simulink model "simulation_environment_thesis"  (R2024b)
settings: Solver=VariableStepAuto  StartTime=0.0  StopTime=3200  RelTol=1e-3
values from neighbouring files (symbols this model uses):
  A = [1, dt; 0, 1]    [estimate_and_control_fully_actuated_with_current.m]
  A1 = [1, 0, 0, 0, dt, 0 ... (first row only)    [estimate_and_control_fully_actuated_with_current.m]
  alpha = 10    [estimate_and_control_fully_actuated_with_current.m]
  alpha_xy_1 = 1    [estimate_and_control_fully_actuated_with_current.m]
  alpha_z_1 = 5    [estimate_and_control_fully_actuated_with_current.m]
  Az1 = [1, dt ... (first row only)    [estimate_and_control_fully_actuated_with_current.m]
  B = [dt; 0]    [estimate_and_control_fully_actuated_with_current.m]
  B1 = [dt, 0 ... (first row only)    [estimate_and_control_fully_actuated_with_current.m]
  Bz1 = [dt ... (first row only)    [estimate_and_control_fully_actuated_with_current.m]
  C1 = [1, 0, 0, 0, 0, 0 ... (first row only)    [estimate_and_control_fully_actuated_with_current.m]
  circumference_x = min_dist_from_ds*cos(theta)    [estimate_and_control_fully_actuated_with_current.m]
  circumference_y = min_dist_from_ds*sin(theta)    [estimate_and_control_fully_actuated_with_current.m]
  const_current = [-r; -s; 0; 0]    [estimate_and_control_fully_actuated_with_current.m]
  csi_psi_1 = 0.0003    [estimate_and_control_fully_actuated_with_current.m]
  csi_r_1 = 0.0003    [estimate_and_control_fully_actuated_with_current.m]
  csi_vcx_1 = 0.0003    [estimate_and_control_fully_actuated_with_current.m]
  csi_vcy_1 = 0.0003    [estimate_and_control_fully_actuated_with_current.m]
  csi_vcz_1 = 0.005    [estimate_and_control_fully_actuated_with_current.m]
  csi_x_1 = 0.003    [estimate_and_control_fully_actuated_with_current.m]
  csi_y_1 = 0.003    [estimate_and_control_fully_actuated_with_current.m]
  csi_z_1 = 0.005    [estimate_and_control_fully_actuated_with_current.m]
  Cz1 = [1, 0]    [estimate_and_control_fully_actuated_with_current.m]
  d = 1    [estimate_and_control_fully_actuated_with_current.m]
  delta_FOV = pi/7    (= 0.448798950513)    [estimate_and_control_fully_actuated_with_current.m]
  desired_speed = speed_0    [estimate_and_control_fully_actuated_with_current.m]
  dt = 1/Tsim    (= 0.005)    [estimate_and_control_fully_actuated_with_current.m]
  eta1 = deg2rad(0.5)    [estimate_and_control_fully_actuated_with_current.m]
  eta2 = deg2rad(0.5)    [estimate_and_control_fully_actuated_with_current.m]
  eta_psi_1 = 0.003    [estimate_and_control_fully_actuated_with_current.m]
  eta_x_1 = 0.03    [estimate_and_control_fully_actuated_with_current.m]
  eta_y_1 = 0.03    [estimate_and_control_fully_actuated_with_current.m]
  eta_z_1 = 0.005    [estimate_and_control_fully_actuated_with_current.m]
  G = dt*eye(2)    [estimate_and_control_fully_actuated_with_current.m]
  G1 = dt*eye(6)    [estimate_and_control_fully_actuated_with_current.m]
  Gz1 = dt*eye(2)    [estimate_and_control_fully_actuated_with_current.m]
  left_reference = L_x*[cos(NED_psi_ds); sin(NED_psi_ds); 0] + L_y_l*[-sin(NED_psi_ds); cos(NED_psi_ds); 0]    [estimate_and_control_fully_actuated_with_current.m]
  max_dist_from_ship = 60    [estimate_and_control_fully_actuated_with_current.m]
  min_dist_from_ds = 45    [estimate_and_control_fully_actuated_with_current.m]
  mu = -5    [estimate_and_control_fully_actuated_with_current.m]
  NED_psi_ds = -pi/2    (= -1.57079632679)    [estimate_and_control_fully_actuated_with_current.m]
  p_b1 = [-90; 90; 5]    [estimate_and_control_fully_actuated_with_current.m]
  position_t_0 = [0; 0; 0; 0]    [estimate_and_control_fully_actuated_with_current.m]
  psi_P = linspace(0,2*pi,360)'    [estimate_and_control_fully_actuated_with_current.m]
  Q = [csi1_kf^2, 0 ... (first row only)    [estimate_and_control_fully_actuated_with_current.m]
  Q1 = [csi_x_1_kf^2, 0, 0, 0, 0, 0 ... (first row only)    [estimate_and_control_fully_actuated_with_current.m]
  Qz1 = [csi_z_1_kf^2, 0 ... (first row only)    [estimate_and_control_fully_actuated_with_current.m]
  R = [eta1_kf^2, 0 ... (first row only)    [estimate_and_control_fully_actuated_with_current.m]
  R1 = [eta_x_1_kf^2, 0, 0 ... (first row only)    [estimate_and_control_fully_actuated_with_current.m]
  right_reference = L_x*[cos(NED_psi_ds); sin(NED_psi_ds); 0] + L_y_r*[sin(NED_psi_ds); -cos(NED_psi_ds); 0]    [estimate_and_control_fully_actuated_with_current.m]
  Rz1 = eta_z_1_kf^2    (= 1e-06)    [estimate_and_control_fully_actuated_with_current.m]
  speed_0 = [0.1, 0.1, 0, 0]'    [estimate_and_control_fully_actuated_with_current.m]
  tau_heave_sat = 30    [estimate_and_control_fully_actuated_with_current.m]
  tau_surge_sat = 10    [estimate_and_control_fully_actuated_with_current.m]
  tau_sway_sat = 30    [estimate_and_control_fully_actuated_with_current.m]
  tau_yaw_sat = 0.1    [estimate_and_control_fully_actuated_with_current.m]
  var_vm_x = 0.001    [estimate_and_control_fully_actuated_with_current.m]
  var_vm_y = 0.001    [estimate_and_control_fully_actuated_with_current.m]
  var_vm_z = 0.001    [estimate_and_control_fully_actuated_with_current.m]
undefined symbols (not defined in the file or in its folder): A2, alpha2, B2, csi1_2, csi2_2, csi3_2, csi4_2, csi5_2, csi6_2, csi7_2, csi8_2, eta1_2, eta2_2, eta3_2, eta4_2, eta5_2, eta6_2, G2, Q2, R2
libraries: simulink x60, simulink_extras x5
(part 5 of 16)

== system simulation_environment_thesis/PHASE 2.21/dynamic + kinematic models4 ==
  note: body frame
  note: ds frame
  note: body frame
  tau : Inport
  ref speed : Inport  Port=2
  last_yaw__from_ship : Inport  Port=3
  yaw_est : Inport  Port=4
  ds_p_auv : Inport  Port=5
  Constant : Constant  Value=0
  Demux : Demux
  Integrator1 : Integrator  InitialConditionSource=external
  Integrator2 : Integrator  InitialConditionSource=external
  Integrator4 : Integrator  InitialCondition=speed_0  UpperSaturationLimit=10^3  LowerSaturationLimit=-10^3
  Integrator5 : Integrator  InitialCondition=const_current
  MATLAB Function : MATLAB Function  TreatAsAtomicUnit=on
  MATLAB Function7 : MATLAB Function  TreatAsAtomicUnit=on
  MATLAB Function9 : MATLAB Function  TreatAsAtomicUnit=on
  Mux : Mux  Inputs=2
  Mux1 : Mux  Inputs=2
  Mux3 : Mux  Inputs=3
  Mux4 : Mux
  Random Number : RandomNumber  Variance=csi1_2^2  Seed=290635734  SampleTime=dt
  Random Number1 : RandomNumber  Variance=csi3_2^2  Seed=1610892866  SampleTime=dt
  Random Number10 : RandomNumber  Variance=csi8_2^2  Seed=308002504  SampleTime=dt
  Random Number3 : RandomNumber  Variance=csi6_2^2  Seed=137035395  SampleTime=dt
  Random Number4 : RandomNumber  Variance=csi4_2^2  Seed=690147537  SampleTime=dt
  Random Number5 : RandomNumber  Variance=csi2_2^2  Seed=1826908498  SampleTime=dt
  Random Number8 : RandomNumber  Variance=csi5_2^2  Seed=1139063427  SampleTime=dt
  Random Number9 : RandomNumber  Variance=csi7_2^2  Seed=54081086  SampleTime=dt
  Selector1 : Selector  InputPortWidth=4  IndexOptions=Index vector (dialog)  Indices=[4]  OutputSizes=1
  Selector2 : Selector  InputPortWidth=4  IndexOptions=Index vector (dialog)  Indices=[1 2 3]  OutputSizes=1
  Selector3 : Selector  InputPortWidth=4  IndexOptions=Index vector (dialog)  Indices=[1 2 3]  OutputSizes=1
  Sum{,1..2,5} (4 alike) : Sum  Inputs=|++
  Sum4 : Sum  Inputs=|+-
  error speed : Outport
  v_tot ds : Outport  Port=2
  p : Outport  Port=3
  yaw : Outport  Port=4
  body speed : Outport  Port=5
  wires:
  ref speed/1 -> Sum4/1
  MATLAB Function9/1(eta_dot) -> Sum5/1, Demux/1
  tau/1 -> MATLAB Function7/2(tau)
  Sum4/1 -> error speed/1
  Sum5/1 -> Selector3/1, v_tot ds/1
  Integrator4/1 -> MATLAB Function7/1(speed), Sum4/2, body speed/1, MATLAB Function9/2(speed)
  Integrator5/1 -> Sum5/2
  last_yaw__from_ship/1 -> Integrator1/2
  Integrator1/1 -> MATLAB Function/1("angle ")
  yaw_est/1 -> MATLAB Function9/1(yaw)
  MATLAB Function/1(angle) -> Mux/2, yaw/1
  ds_p_auv/1 -> Integrator2/2
  Random Number1/1 -> Mux3/3
  Mux3/1 -> Sum/2
  Sum/1 -> Integrator2/1
  Random Number5/1 -> Mux3/2
  Random Number/1 -> Mux3/1
  Integrator2/1 -> Mux/1
  Random Number10/1 -> Sum2/2
  Selector1/1 -> Sum2/1
  Selector2/1 -> Mux1/1
  Sum2/1 -> Mux1/2
  Mux1/1 -> Integrator4/1
  MATLAB Function7/1(speed_dot) -> Selector2/1, Selector1/1
  Random Number9/1 -> Sum1/2
  Sum1/1 -> Integrator1/1
  Demux/4 -> Sum1/1
  Random Number8/1 -> Mux4/2
  Random Number4/1 -> Mux4/1
  Mux4/1 -> Integrator5/1
  Random Number3/1 -> Mux4/3
  Selector3/1 -> Sum/1
  Mux/1 -> p/1
  Constant/1 -> Mux4/4

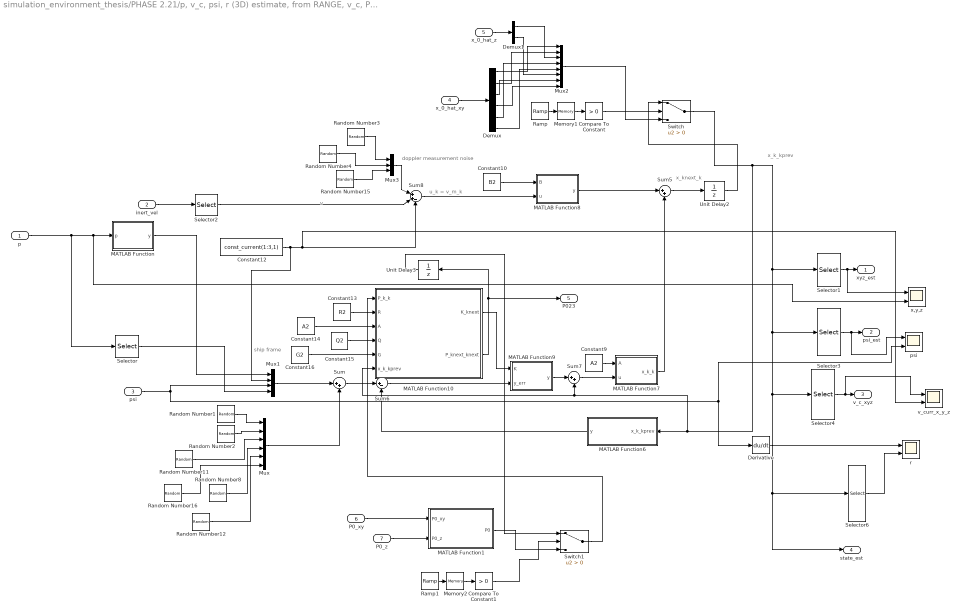
== system simulation_environment_thesis/PHASE 2.21/p, v_c, psi, r (3D) estimate, from RANGE, v_c, PSI measurement ==
  note: x_k_kprev
  note: x_knext_k
  note: ship frame
  note: v
  note: doppler measurement noise
  note: u_k = v_m_k
  p : Inport
  inert_vel : Inport  Port=2
  psi : Inport  Port=3
  x_0_hat_xy : Inport  Port=4
  x_0_hat_z : Inport  Port=5
  P0_xy : Inport  Port=6
  P0_z : Inport  Port=7
  Compare To Constant : simulink/Logic and Bit Operations/Compare To Constant  relop=>  const=0  OutDataTypeStr=boolean
  Compare To Constant1 : simulink/Logic and Bit Operations/Compare To Constant  relop=>  const=0  OutDataTypeStr=boolean
  Constant10 : Constant  Value=B2
  Constant12 : Constant  Value=const_current(1:3,1)  VectorParams1D=off
  Constant13 : Constant  Value=R2
  Constant14 : Constant  Value=A2
  Constant15 : Constant  Value=Q2
  Constant16 : Constant  Value=G2
  Constant9 : Constant  Value=A2  VectorParams1D=off
  Demux : Demux  Outputs=6
  Demux1 : Demux  Outputs=2
  Derivative : Derivative
  MATLAB Function : MATLAB Function  TreatAsAtomicUnit=on
  MATLAB Function1 : MATLAB Function  TreatAsAtomicUnit=on
  MATLAB Function10 : MATLAB Function  TreatAsAtomicUnit=on
  MATLAB Function6 : MATLAB Function  TreatAsAtomicUnit=on
  MATLAB Function7 : MATLAB Function  TreatAsAtomicUnit=on
  MATLAB Function8 : MATLAB Function  TreatAsAtomicUnit=on
  MATLAB Function9 : MATLAB Function  TreatAsAtomicUnit=on
  Memory1 : Memory
  Memory2 : Memory
  Mux : Mux  Inputs=6
  Mux1 : Mux
  Mux2 : Mux  Inputs=8
  Mux3 : Mux  Inputs=3
  Ramp : simulink/Sources/Ramp  slope=1  start=0  InitialOutput=0
  Ramp1 : simulink/Sources/Ramp  slope=1  start=0  InitialOutput=0
  Random Number1 : RandomNumber  Variance=eta1_2^2  Seed=1592011725  SampleTime=dt
  Random Number11 : RandomNumber  Variance=eta3_2^2  Seed=403145296  SampleTime=dt
  Random Number12 : RandomNumber  Variance=eta5_2^2  Seed=421479706  SampleTime=dt
  Random Number15 : RandomNumber  Variance=var_vm_z^2  Seed=536323856  SampleTime=dt
  Random Number16 : RandomNumber  Variance=eta6_2^2  Seed=285935395  SampleTime=dt
  Random Number2 : RandomNumber  Variance=eta2_2^2  Seed=1745211012  SampleTime=dt
  Random Number3 : RandomNumber  Variance=var_vm_x^2  Seed=980211847  SampleTime=dt
  Random Number4 : RandomNumber  Variance=var_vm_y^2  Seed=1399358789  SampleTime=dt
  Random Number8 : RandomNumber  Variance=eta4_2^2  Seed=2011049955  SampleTime=dt
  Selector : Selector  InputPortWidth=3  IndexOptions=Index vector (dialog)  Indices=[3]  OutputSizes=1
  Selector1 : Selector  InputPortWidth=8  IndexOptions=Index vector (dialog)  Indices=[1 2 3]  OutputSizes=1
  Selector2 : Selector  InputPortWidth=4  IndexOptions=Index vector (dialog)  Indices=[1 2 3]  OutputSizes=1
  Selector3 : Selector  InputPortWidth=8  IndexOptions=Index vector (dialog)  Indices=[7]  OutputSizes=1
  Selector4 : Selector  InputPortWidth=8  IndexOptions=Index vector (dialog)  Indices=[4 5 6]  OutputSizes=1
  Selector6 : Selector  InputPortWidth=8  IndexOptions=Index vector (dialog)  Indices=[8]  OutputSizes=1
  Sum : Sum  Inputs=|++
  Sum5 : Sum  Inputs=|++
  Sum6 : Sum  Inputs=|+-
  Sum7 : Sum  Inputs=|++
  Sum8 : Sum  Inputs=|++-
  Switch : Switch  Criteria=u2 > Threshold  SaturateOnIntegerOverflow=off  InputSameDT=off
  Switch1 : Switch  Criteria=u2 > Threshold  SaturateOnIntegerOverflow=off  InputSameDT=off
  Unit Delay2 : UnitDelay  SampleTime=-1  HasFrameUpgradeWarning=on
  Unit Delay3 : UnitDelay  InitialCondition=alpha2*eye(8)  SampleTime=-1  HasFrameUpgradeWarning=on
  "psi " : Scope  NumInputPorts=2
  r : Scope  NumInputPorts=2
  v_curr_x_y_z : Scope  NumInputPorts=2
  x,y,z : Scope  NumInputPorts=2
  xyz_est : Outport
  psi_est : Outport  Port=2
  v_c_xyz : Outport  Port=3
  state_est : Outport  Port=4
  P023 : Outport  Port=5
  wires:
  MATLAB Function7/1(x_k_k) -> Sum5/2
  Constant14/1 -> MATLAB Function10/3(A)
  Sum7/1 -> MATLAB Function7/2(u)
  Random Number4/1 -> Mux3/2
  MATLAB Function8/1(y) -> Sum5/1
  Random Number3/1 -> Mux3/1
  Constant15/1 -> MATLAB Function10/4(Q)
  MATLAB Function9/1(y) -> Sum7/1
  Sum5/1 -> Unit Delay2/1
  MATLAB Function10/2(P_knext_knext) -> P023/1, Unit Delay3/1
  Sum8/1 -> MATLAB Function8/2(u)
  MATLAB Function10/1(K_knext) -> MATLAB Function9/1(K)
  Constant9/1 -> MATLAB Function7/1(A)
  Constant13/1 -> MATLAB Function10/2(R)
  Sum6/1 -> MATLAB Function9/2(y_err)
  Constant10/1 -> MATLAB Function8/1(B)
  Mux3/1 -> Sum8/1
  Constant16/1 -> MATLAB Function10/5(G)
  inert_vel/1 -> Selector2/1
  Sum/1 -> Sum6/1
  MATLAB Function6/1(y) -> Sum6/2
  Selector1/1 -> x,y,z/1, xyz_est/1
  psi/1 -> "psi "/2, Derivative/1, Mux1/3
  Mux1/1 -> Sum/1
  Selector3/1 -> "psi "/1, psi_est/1
  Selector4/1 -> v_curr_x_y_z/1, v_c_xyz/1
  Selector2/1 -> Sum8/2
  Mux/1 -> Sum/2
  Random Number2/1 -> Mux/2
  Random Number11/1 -> Mux/3
  Random Number1/1 -> Mux/1
  MATLAB Function/1(y) -> Mux1/1
  Constant12/1 -> Mux1/2, Sum8/3, v_curr_x_y_z/2
  Random Number8/1 -> Mux/4
  Random Number12/1 -> Mux/5
  Derivative/1 -> r/1
  Selector6/1 -> r/2
  Random Number15/1 -> Mux3/3
  Random Number16/1 -> Mux/6
  Selector/1 -> Mux1/4
  p/1 -> Selector/1, x,y,z/2, MATLAB Function/1(p)
  Switch/1 -> Selector3/1, Selector4/1, state_est/1, Selector6/1, Selector1/1, MATLAB Function10/6(x_k_kprev), Sum7/2, MATLAB Function6/1(x_k_kprev)
  Memory1/1 -> Compare To Constant/1
  Ramp/1 -> Memory1/1
  Compare To Constant/1 -> Switch/2
  Unit Delay2/1 -> Switch/1
  x_0_hat_z/1 -> Demux1/1
  x_0_hat_xy/1 -> Demux/1
  Demux/1 -> Mux2/1
  Demux/2 -> Mux2/2
  Demux1/1 -> Mux2/3
  Demux/5 -> Mux2/4
  Demux/6 -> Mux2/5
  Demux1/2 -> Mux2/6
  Demux/3 -> Mux2/7
  Demux/4 -> Mux2/8
  Mux2/1 -> Switch/3
  P0_xy/1 -> MATLAB Function1/1(P0_xy)
  P0_z/1 -> MATLAB Function1/2(P0_z)
  Ramp1/1 -> Memory2/1
  Memory2/1 -> Compare To Constant1/1
  Compare To Constant1/1 -> Switch1/2
  MATLAB Function1/1(P0) -> Switch1/3
  Unit Delay3/1 -> Switch1/1
  Switch1/1 -> MATLAB Function10/1(P_k_k)

== system simulation_environment_thesis/PHASE 2.22/CONTROLLER4 ==
  v_err : Inport
  psi_err : Inport  Port=2
  speed_0 : Inport  Port=3
  z_err : Inport  Port=4
  ANTIWINDUP SURGE : Subsystem
  ANTIWINDUP SWAY : Subsystem
  ANTIWINDUP_HEAVE : Subsystem
  ANTIWINDUP_YAW : Subsystem
  Demux : Demux
  MATLAB Function3 : MATLAB Function  TreatAsAtomicUnit=on
  Mux : Mux
  tau : Outport
  wires:
  MATLAB Function3/12(K_i_psi) -> ANTIWINDUP_YAW/1(K1)
  ANTIWINDUP SURGE/1(tau_u) -> Mux/1
  ANTIWINDUP_HEAVE/1(tau_w) -> Mux/3
  ANTIWINDUP_YAW/1(tau_psi) -> Mux/4
  MATLAB Function3/1(K_i_u) -> ANTIWINDUP SURGE/2(K)
  MATLAB Function3/3(K_t_u) -> ANTIWINDUP SURGE/4(K_t_u)
  MATLAB Function3/2(K_p_u) -> ANTIWINDUP SURGE/3(K1)
  speed_0/1 -> MATLAB Function3/1(speed_0)
  Demux/1 -> ANTIWINDUP SURGE/1(in)
  MATLAB Function3/14(K_t_psi) -> ANTIWINDUP_YAW/3(K_t_psi)
  MATLAB Function3/11(K_p_psi) -> ANTIWINDUP_YAW/2(K2)
  ANTIWINDUP SWAY/1(tau_v) -> Mux/2
  v_err/1 -> Demux/1
  Mux/1 -> tau/1
  psi_err/1 -> ANTIWINDUP_YAW/4(in1)
  Demux/2 -> ANTIWINDUP SWAY/1(in)
  MATLAB Function3/4(K_i_v) -> ANTIWINDUP SWAY/2(K)
  MATLAB Function3/5(K_p_v) -> ANTIWINDUP SWAY/3(K1)
  MATLAB Function3/6(K_t_v) -> ANTIWINDUP SWAY/4(K_t_v)
  MATLAB Function3/13(K_d_psi) -> ANTIWINDUP_YAW/6(K)
  Demux/4 -> ANTIWINDUP_YAW/5(in)
  MATLAB Function3/7(K_i_w) -> ANTIWINDUP_HEAVE/1(K1)
  MATLAB Function3/8(K_p_w) -> ANTIWINDUP_HEAVE/2(K2)
  MATLAB Function3/9(K_d_w) -> ANTIWINDUP_HEAVE/6(K)
  MATLAB Function3/10(K_t_w) -> ANTIWINDUP_HEAVE/3(K_t_w)
  Demux/3 -> ANTIWINDUP_HEAVE/5(in)
  z_err/1 -> ANTIWINDUP_HEAVE/4(in1)

== system simulation_environment_thesis/PHASE 2.22/dynamic + kinematic models1 ==
  note: body frame
  note: ds frame
  note: body frame
  tau : Inport
  ref speed : Inport  Port=2
  last_yaw__from_ship : Inport  Port=3
  yaw_est : Inport  Port=4
  ds_p_auv : Inport  Port=5
  Constant : Constant  Value=0
  Demux : Demux
  Integrator1 : Integrator  InitialConditionSource=external
  Integrator2 : Integrator  InitialConditionSource=external
  Integrator4 : Integrator  InitialCondition=speed_0  UpperSaturationLimit=10^3  LowerSaturationLimit=-10^3
  Integrator5 : Integrator  InitialCondition=const_current
  MATLAB Function : MATLAB Function  TreatAsAtomicUnit=on
  MATLAB Function7 : MATLAB Function  TreatAsAtomicUnit=on
  MATLAB Function9 : MATLAB Function  TreatAsAtomicUnit=on
  Mux : Mux  Inputs=2
  Mux1 : Mux  Inputs=2
  Mux3 : Mux  Inputs=3
  Mux4 : Mux
  Random Number : RandomNumber  Variance=csi1_2^2  Seed=1280987953  SampleTime=dt
  Random Number1 : RandomNumber  Variance=csi3_2^2  Seed=123915637  SampleTime=dt
  Random Number10 : RandomNumber  Variance=csi8_2^2  Seed=337781319  SampleTime=dt
  Random Number3 : RandomNumber  Variance=csi6_2^2  Seed=1365143274  SampleTime=dt
  Random Number4 : RandomNumber  Variance=csi4_2^2  Seed=894166265  SampleTime=dt
  Random Number5 : RandomNumber  Variance=csi2_2^2  Seed=538465485  SampleTime=dt
  Random Number8 : RandomNumber  Variance=csi5_2^2  Seed=774913930  SampleTime=dt
  Random Number9 : RandomNumber  Variance=csi7_2^2  Seed=364931984  SampleTime=dt
  Selector1 : Selector  InputPortWidth=4  IndexOptions=Index vector (dialog)  Indices=[4]  OutputSizes=1
  Selector2 : Selector  InputPortWidth=4  IndexOptions=Index vector (dialog)  Indices=[1 2 3]  OutputSizes=1
  Selector3 : Selector  InputPortWidth=4  IndexOptions=Index vector (dialog)  Indices=[1 2 3]  OutputSizes=1
  Sum{,1..2,5} (4 alike) : Sum  Inputs=|++
  Sum4 : Sum  Inputs=|+-
  error speed : Outport
  v_tot ds : Outport  Port=2
  p : Outport  Port=3
  yaw : Outport  Port=4
  body speed : Outport  Port=5
  wires:
  ref speed/1 -> Sum4/1
  MATLAB Function9/1(eta_dot) -> Sum5/1, Demux/1
  tau/1 -> MATLAB Function7/2(tau)
  Sum4/1 -> error speed/1
  Sum5/1 -> Selector3/1, v_tot ds/1
  Integrator4/1 -> MATLAB Function7/1(speed), Sum4/2, body speed/1, MATLAB Function9/2(speed)
  Integrator5/1 -> Sum5/2
  last_yaw__from_ship/1 -> Integrator1/2
  Integrator1/1 -> MATLAB Function/1("angle ")
  yaw_est/1 -> MATLAB Function9/1(yaw)
  MATLAB Function/1(angle) -> Mux/2, yaw/1
  ds_p_auv/1 -> Integrator2/2
  Random Number1/1 -> Mux3/3
  Mux3/1 -> Sum/2
  Sum/1 -> Integrator2/1
  Random Number5/1 -> Mux3/2
  Random Number/1 -> Mux3/1
  Integrator2/1 -> Mux/1
  Random Number10/1 -> Sum2/2
  Selector1/1 -> Sum2/1
  Selector2/1 -> Mux1/1
  Sum2/1 -> Mux1/2
  Mux1/1 -> Integrator4/1
  MATLAB Function7/1(speed_dot) -> Selector2/1, Selector1/1
  Random Number9/1 -> Sum1/2
  Sum1/1 -> Integrator1/1
  Demux/4 -> Sum1/1
  Random Number8/1 -> Mux4/2
  Random Number4/1 -> Mux4/1
  Mux4/1 -> Integrator5/1
  Random Number3/1 -> Mux4/3
  Selector3/1 -> Sum/1
  Mux/1 -> p/1
  Constant/1 -> Mux4/4

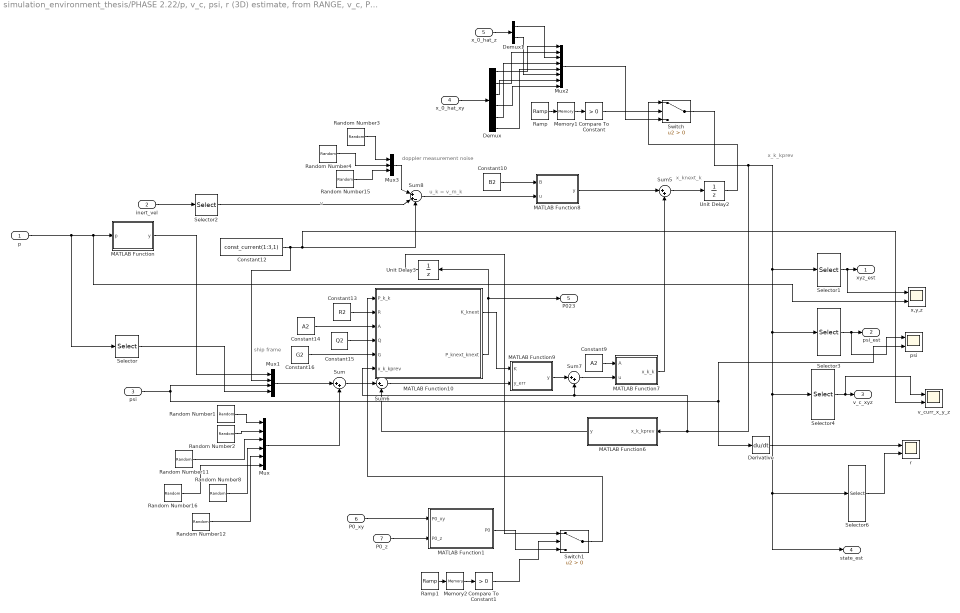
== system simulation_environment_thesis/PHASE 2.22/p, v_c, psi, r (3D) estimate, from RANGE, v_c, PSI measurement ==
  note: x_k_kprev
  note: x_knext_k
  note: ship frame
  note: v
  note: doppler measurement noise
  note: u_k = v_m_k
  p : Inport
  inert_vel : Inport  Port=2
  psi : Inport  Port=3
  x_0_hat_xy : Inport  Port=4
  x_0_hat_z : Inport  Port=5
  P0_xy : Inport  Port=6
  P0_z : Inport  Port=7
  Compare To Constant : simulink/Logic and Bit Operations/Compare To Constant  relop=>  const=0  OutDataTypeStr=boolean
  Compare To Constant1 : simulink/Logic and Bit Operations/Compare To Constant  relop=>  const=0  OutDataTypeStr=boolean
  Constant10 : Constant  Value=B2
  Constant12 : Constant  Value=const_current(1:3,1)  VectorParams1D=off
  Constant13 : Constant  Value=R2
  Constant14 : Constant  Value=A2
  Constant15 : Constant  Value=Q2
  Constant16 : Constant  Value=G2
  Constant9 : Constant  Value=A2  VectorParams1D=off
  Demux : Demux  Outputs=6
  Demux1 : Demux  Outputs=2
  Derivative : Derivative
  MATLAB Function : MATLAB Function  TreatAsAtomicUnit=on
  MATLAB Function1 : MATLAB Function  TreatAsAtomicUnit=on
  MATLAB Function10 : MATLAB Function  TreatAsAtomicUnit=on
  MATLAB Function6 : MATLAB Function  TreatAsAtomicUnit=on
  MATLAB Function7 : MATLAB Function  TreatAsAtomicUnit=on
  MATLAB Function8 : MATLAB Function  TreatAsAtomicUnit=on
  MATLAB Function9 : MATLAB Function  TreatAsAtomicUnit=on
  Memory1 : Memory
  Memory2 : Memory
  Mux : Mux  Inputs=6
  Mux1 : Mux
  Mux2 : Mux  Inputs=8
  Mux3 : Mux  Inputs=3
  Ramp : simulink/Sources/Ramp  slope=1  start=0  InitialOutput=0
  Ramp1 : simulink/Sources/Ramp  slope=1  start=0  InitialOutput=0
  Random Number1 : RandomNumber  Variance=eta1_2^2  Seed=1089217264  SampleTime=dt
  Random Number11 : RandomNumber  Variance=eta3_2^2  Seed=72035191  SampleTime=dt
  Random Number12 : RandomNumber  Variance=eta5_2^2  Seed=2079342519  SampleTime=dt
  Random Number15 : RandomNumber  Variance=var_vm_z^2  Seed=110850037  SampleTime=dt
  Random Number16 : RandomNumber  Variance=eta6_2^2  Seed=1575761613  SampleTime=dt
  Random Number2 : RandomNumber  Variance=eta2_2^2  Seed=1411597288  SampleTime=dt
  Random Number3 : RandomNumber  Variance=var_vm_x^2  Seed=1818164200  SampleTime=dt
  Random Number4 : RandomNumber  Variance=var_vm_y^2  Seed=1838379340  SampleTime=dt
  Random Number8 : RandomNumber  Variance=eta4_2^2  Seed=1668214198  SampleTime=dt
  Selector : Selector  InputPortWidth=3  IndexOptions=Index vector (dialog)  Indices=[3]  OutputSizes=1
  Selector1 : Selector  InputPortWidth=8  IndexOptions=Index vector (dialog)  Indices=[1 2 3]  OutputSizes=1
  Selector2 : Selector  InputPortWidth=4  IndexOptions=Index vector (dialog)  Indices=[1 2 3]  OutputSizes=1
  Selector3 : Selector  InputPortWidth=8  IndexOptions=Index vector (dialog)  Indices=[7]  OutputSizes=1
  Selector4 : Selector  InputPortWidth=8  IndexOptions=Index vector (dialog)  Indices=[4 5 6]  OutputSizes=1
  Selector6 : Selector  InputPortWidth=8  IndexOptions=Index vector (dialog)  Indices=[8]  OutputSizes=1
  Sum : Sum  Inputs=|++
  Sum5 : Sum  Inputs=|++
  Sum6 : Sum  Inputs=|+-
  Sum7 : Sum  Inputs=|++
  Sum8 : Sum  Inputs=|++-
  Switch : Switch  Criteria=u2 > Threshold  SaturateOnIntegerOverflow=off  InputSameDT=off
  Switch1 : Switch  Criteria=u2 > Threshold  SaturateOnIntegerOverflow=off  InputSameDT=off
  Unit Delay2 : UnitDelay  SampleTime=-1  HasFrameUpgradeWarning=on
  Unit Delay3 : UnitDelay  InitialCondition=alpha2*eye(8)  SampleTime=-1  HasFrameUpgradeWarning=on
  "psi " : Scope  NumInputPorts=2
  r : Scope  NumInputPorts=2
  v_curr_x_y_z : Scope  NumInputPorts=2
  x,y,z : Scope  NumInputPorts=2
  xyz_est : Outport
  psi_est : Outport  Port=2
  v_c_xyz : Outport  Port=3
  state_est : Outport  Port=4
  P023 : Outport  Port=5
  wires:
  MATLAB Function7/1(x_k_k) -> Sum5/2
  Constant14/1 -> MATLAB Function10/3(A)
  Sum7/1 -> MATLAB Function7/2(u)
  Random Number4/1 -> Mux3/2
  MATLAB Function8/1(y) -> Sum5/1
  Random Number3/1 -> Mux3/1
  Constant15/1 -> MATLAB Function10/4(Q)
  MATLAB Function9/1(y) -> Sum7/1
  Sum5/1 -> Unit Delay2/1
  MATLAB Function10/2(P_knext_knext) -> P023/1, Unit Delay3/1
  Sum8/1 -> MATLAB Function8/2(u)
  MATLAB Function10/1(K_knext) -> MATLAB Function9/1(K)
  Constant9/1 -> MATLAB Function7/1(A)
  Constant13/1 -> MATLAB Function10/2(R)
  Sum6/1 -> MATLAB Function9/2(y_err)
  Constant10/1 -> MATLAB Function8/1(B)
  Mux3/1 -> Sum8/1
  Constant16/1 -> MATLAB Function10/5(G)
  inert_vel/1 -> Selector2/1
  Sum/1 -> Sum6/1
  MATLAB Function6/1(y) -> Sum6/2
  Selector1/1 -> x,y,z/1, xyz_est/1
  psi/1 -> "psi "/2, Derivative/1, Mux1/3
  Mux1/1 -> Sum/1
  Selector3/1 -> "psi "/1, psi_est/1
  Selector4/1 -> v_curr_x_y_z/1, v_c_xyz/1
  Selector2/1 -> Sum8/2
  Mux/1 -> Sum/2
  Random Number2/1 -> Mux/2
  Random Number11/1 -> Mux/3
  Random Number1/1 -> Mux/1
  MATLAB Function/1(y) -> Mux1/1
  Constant12/1 -> Mux1/2, Sum8/3, v_curr_x_y_z/2
  Random Number8/1 -> Mux/4
  Random Number12/1 -> Mux/5
  Derivative/1 -> r/1
  Selector6/1 -> r/2
  Random Number15/1 -> Mux3/3
  Random Number16/1 -> Mux/6
  Selector/1 -> Mux1/4
  p/1 -> Selector/1, x,y,z/2, MATLAB Function/1(p)
  Switch/1 -> Selector3/1, Selector4/1, state_est/1, Selector6/1, Selector1/1, Sum7/2, MATLAB Function10/6(x_k_kprev), MATLAB Function6/1(x_k_kprev)
  Memory1/1 -> Compare To Constant/1
  Ramp/1 -> Memory1/1
  Compare To Constant/1 -> Switch/2
  Unit Delay2/1 -> Switch/1
  x_0_hat_z/1 -> Demux1/1
  x_0_hat_xy/1 -> Demux/1
  Demux/1 -> Mux2/1
  Demux/2 -> Mux2/2
  Demux1/1 -> Mux2/3
  Demux/5 -> Mux2/4
  Demux/6 -> Mux2/5
  Demux1/2 -> Mux2/6
  Demux/3 -> Mux2/7
  Demux/4 -> Mux2/8
  Mux2/1 -> Switch/3
  P0_xy/1 -> MATLAB Function1/1(P0_xy)
  P0_z/1 -> MATLAB Function1/2(P0_z)
  Ramp1/1 -> Memory2/1
  Memory2/1 -> Compare To Constant1/1
  Compare To Constant1/1 -> Switch1/2
  MATLAB Function1/1(P0) -> Switch1/3
  Unit Delay3/1 -> Switch1/1
  Switch1/1 -> MATLAB Function10/1(P_k_k)

== system simulation_environment_thesis/PHASE 2.24/CONTROLLER4 ==
  v_err : Inport
  psi_err : Inport  Port=2
  speed_0 : Inport  Port=3
  z_err : Inport  Port=4
  ANTIWINDUP SURGE : Subsystem
  ANTIWINDUP SWAY : Subsystem
  ANTIWINDUP_HEAVE : Subsystem
  ANTIWINDUP_YAW : Subsystem
  Demux : Demux
  MATLAB Function3 : MATLAB Function  TreatAsAtomicUnit=on
  Mux : Mux
  tau : Outport
  wires:
  MATLAB Function3/12(K_i_psi) -> ANTIWINDUP_YAW/1(K1)
  ANTIWINDUP SURGE/1(tau_u) -> Mux/1
  ANTIWINDUP_HEAVE/1(tau_w) -> Mux/3
  ANTIWINDUP_YAW/1(tau_psi) -> Mux/4
  MATLAB Function3/1(K_i_u) -> ANTIWINDUP SURGE/2(K)
  MATLAB Function3/3(K_t_u) -> ANTIWINDUP SURGE/4(K_t_u)
  MATLAB Function3/2(K_p_u) -> ANTIWINDUP SURGE/3(K1)
  speed_0/1 -> MATLAB Function3/1(speed_0)
  Demux/1 -> ANTIWINDUP SURGE/1(in)
  MATLAB Function3/14(K_t_psi) -> ANTIWINDUP_YAW/3(K_t_psi)
  MATLAB Function3/11(K_p_psi) -> ANTIWINDUP_YAW/2(K2)
  ANTIWINDUP SWAY/1(tau_v) -> Mux/2
  v_err/1 -> Demux/1
  Mux/1 -> tau/1
  psi_err/1 -> ANTIWINDUP_YAW/4(in1)
  Demux/2 -> ANTIWINDUP SWAY/1(in)
  MATLAB Function3/4(K_i_v) -> ANTIWINDUP SWAY/2(K)
  MATLAB Function3/5(K_p_v) -> ANTIWINDUP SWAY/3(K1)
  MATLAB Function3/6(K_t_v) -> ANTIWINDUP SWAY/4(K_t_v)
  MATLAB Function3/13(K_d_psi) -> ANTIWINDUP_YAW/6(K)
  Demux/4 -> ANTIWINDUP_YAW/5(in)
  MATLAB Function3/7(K_i_w) -> ANTIWINDUP_HEAVE/1(K1)
  MATLAB Function3/8(K_p_w) -> ANTIWINDUP_HEAVE/2(K2)
  MATLAB Function3/9(K_d_w) -> ANTIWINDUP_HEAVE/6(K)
  MATLAB Function3/10(K_t_w) -> ANTIWINDUP_HEAVE/3(K_t_w)
  Demux/3 -> ANTIWINDUP_HEAVE/5(in)
  z_err/1 -> ANTIWINDUP_HEAVE/4(in1)

== system simulation_environment_thesis/PHASE 2.24/dynamic + kinematic models1 ==
  note: body frame
  note: ds frame
  note: body frame
  tau : Inport
  ref speed : Inport  Port=2
  last_yaw__from_ship : Inport  Port=3
  yaw_est : Inport  Port=4
  ds_p_auv : Inport  Port=5
  Constant : Constant  Value=0
  Demux : Demux
  Integrator1 : Integrator  InitialConditionSource=external
  Integrator2 : Integrator  InitialConditionSource=external
  Integrator4 : Integrator  InitialCondition=speed_0  UpperSaturationLimit=10^3  LowerSaturationLimit=-10^3
  Integrator5 : Integrator  InitialCondition=const_current
  MATLAB Function : MATLAB Function  TreatAsAtomicUnit=on
  MATLAB Function7 : MATLAB Function  TreatAsAtomicUnit=on
  MATLAB Function9 : MATLAB Function  TreatAsAtomicUnit=on
  Mux : Mux  Inputs=2
  Mux1 : Mux  Inputs=2
  Mux3 : Mux  Inputs=3
  Mux4 : Mux
  Random Number : RandomNumber  Variance=csi_x_1^2  Seed=1833923873  SampleTime=dt
  Random Number1 : RandomNumber  Variance=csi_z_1^2  Seed=744874454  SampleTime=dt
  Random Number10 : RandomNumber  Variance=csi_r_1^2  Seed=863987044  SampleTime=dt
  Random Number3 : RandomNumber  Variance=csi_vcz_1^2  Seed=327348082  SampleTime=dt
  Random Number4 : RandomNumber  Variance=csi_vcx_1^2  Seed=1447632064  SampleTime=dt
  Random Number5 : RandomNumber  Variance=csi_y_1^2  Seed=1607275602  SampleTime=dt
  Random Number8 : RandomNumber  Variance=csi_vcy_1^2  Seed=1677010297  SampleTime=dt
  Random Number9 : RandomNumber  Variance=csi_psi_1^2  Seed=1596755842  SampleTime=dt
  Selector1 : Selector  InputPortWidth=4  IndexOptions=Index vector (dialog)  Indices=[4]  OutputSizes=1
  Selector2 : Selector  InputPortWidth=4  IndexOptions=Index vector (dialog)  Indices=[1 2 3]  OutputSizes=1
  Selector3 : Selector  InputPortWidth=4  IndexOptions=Index vector (dialog)  Indices=[1 2 3]  OutputSizes=1
  Sum{,1..2,5} (4 alike) : Sum  Inputs=|++
  Sum4 : Sum  Inputs=|+-
  error speed : Outport
  v_tot ds : Outport  Port=2
  body speed : Outport  Port=3
  p : Outport  Port=4
  yaw : Outport  Port=5
  wires:
  ref speed/1 -> Sum4/1
  MATLAB Function9/1(eta_dot) -> Sum5/1, Demux/1
  tau/1 -> MATLAB Function7/2(tau)
  Sum4/1 -> error speed/1
  Sum5/1 -> Selector3/1, v_tot ds/1
  Integrator4/1 -> MATLAB Function7/1(speed), Sum4/2, body speed/1, MATLAB Function9/2(speed)
  Integrator5/1 -> Sum5/2
  last_yaw__from_ship/1 -> Integrator1/2
  Integrator1/1 -> MATLAB Function/1("angle ")
  yaw_est/1 -> MATLAB Function9/1(yaw)
  MATLAB Function/1(angle) -> Mux/2, yaw/1
  ds_p_auv/1 -> Integrator2/2
  Random Number1/1 -> Mux3/3
  Mux3/1 -> Sum/2
  Sum/1 -> Integrator2/1
  Random Number5/1 -> Mux3/2
  Random Number/1 -> Mux3/1
  Integrator2/1 -> Mux/1
  Random Number10/1 -> Sum2/2
  Selector1/1 -> Sum2/1
  Selector2/1 -> Mux1/1
  Sum2/1 -> Mux1/2
  Mux1/1 -> Integrator4/1
  MATLAB Function7/1(speed_dot) -> Selector2/1, Selector1/1
  Random Number9/1 -> Sum1/2
  Sum1/1 -> Integrator1/1
  Demux/4 -> Sum1/1
  Random Number8/1 -> Mux4/2
  Random Number4/1 -> Mux4/1
  Mux4/1 -> Integrator5/1
  Random Number3/1 -> Mux4/3
  Selector3/1 -> Sum/1
  Mux/1 -> p/1
  Constant/1 -> Mux4/4

== system simulation_environment_thesis/PHASE 2.24/positon and current estimate, XY PLANE from X, Y, psi measurement ==
  note: x_k_kprev
  note: x_knext_k
  note: ship frame
  note: [x
      | y]
  note: v
  note: doppler measurement noise
  note: u_k = v_m_k
  p : Inport
  inert_vel : Inport  Port=2
  psi : Inport  Port=3
  x_0_hat_xy : Inport  Port=4
  P0_xy : Inport  Port=5
  Compare To Constant : simulink/Logic and Bit Operations/Compare To Constant  relop=>  const=0  OutDataTypeStr=boolean
  Compare To Constant1 : simulink/Logic and Bit Operations/Compare To Constant  relop=>  const=0  OutDataTypeStr=boolean
  Constant : Constant  Value=C1
  Constant10 : Constant  Value=B1
  Constant12 : Constant  Value=const_current(1:2,1)  VectorParams1D=off
  Constant13 : Constant  Value=R1
  Constant14 : Constant  Value=A1
  Constant15 : Constant  Value=Q1
  Constant16 : Constant  Value=G1
  Constant9 : Constant  Value=A1  VectorParams1D=off
  Derivative : Derivative
  MATLAB Function10 : MATLAB Function  TreatAsAtomicUnit=on
  MATLAB Function6 : MATLAB Function  TreatAsAtomicUnit=on
  MATLAB Function7 : MATLAB Function  TreatAsAtomicUnit=on
  MATLAB Function8 : MATLAB Function  TreatAsAtomicUnit=on
  MATLAB Function9 : MATLAB Function  TreatAsAtomicUnit=on
  Memory1 : Memory
  Memory2 : Memory
  Mux : Mux  Inputs=3
  Mux1 : Mux  Inputs=2
  Mux3 : Mux  Inputs=2
  Ramp : simulink/Sources/Ramp  slope=1  start=0  InitialOutput=0
  Ramp1 : simulink/Sources/Ramp  slope=1  start=0  InitialOutput=0
  Random Number1 : RandomNumber  Variance=eta_x_1^2  Seed=1100775960  SampleTime=dt
  Random Number2 : RandomNumber  Variance=eta_y_1^2  Seed=1075221843  SampleTime=dt
  Random Number3 : RandomNumber  Variance=var_vm_x^2  Seed=1282275211  SampleTime=dt
  Random Number4 : RandomNumber  Variance=var_vm_y^2  Seed=1450386110  SampleTime=dt
  Random Number8 : RandomNumber  Variance=eta_psi_1^2  Seed=1101695171  SampleTime=dt
  Scope2 : Scope  NumInputPorts=5
  Selector : Selector  InputPortWidth=4  IndexOptions=Index vector (dialog)  Indices=[1 2]  OutputSizes=1
  Selector1 : Selector  InputPortWidth=6  IndexOptions=Index vector (dialog)  Indices=[1 2]  OutputSizes=1
  Selector2 : Selector  InputPortWidth=4  IndexOptions=Index vector (dialog)  Indices=[1 2]  OutputSizes=1
  Selector3 : Selector  InputPortWidth=6  IndexOptions=Index vector (dialog)  Indices=[3]  OutputSizes=1
  Selector4 : Selector  InputPortWidth=6  IndexOptions=Index vector (dialog)  Indices=[5 6]  OutputSizes=1
  Sum : Sum  Inputs=|++
  Sum5 : Sum  Inputs=|++
  Sum6 : Sum  Inputs=|+-
  Sum7 : Sum  Inputs=|++
  Sum8 : Sum  Inputs=|++-
  Switch : Switch  Criteria=u2 > Threshold  SaturateOnIntegerOverflow=off  InputSameDT=off
  Switch1 : Switch  Criteria=u2 > Threshold  SaturateOnIntegerOverflow=off  InputSameDT=off
  Unit Delay2 : UnitDelay  SampleTime=-1  HasFrameUpgradeWarning=on
  Unit Delay3 : UnitDelay  InitialCondition=alpha_xy_1*eye(6)  SampleTime=-1  HasFrameUpgradeWarning=on
  xy_est : Outport
  psi_est : Outport  Port=2
  v_c_xy : Outport  Port=3
  state_est : Outport  Port=4
  P0 : Outport  Port=5
  wires:
  MATLAB Function7/1(x_k_k) -> Sum5/2
  Constant14/1 -> MATLAB Function10/3(A)
  Switch/1 -> Selector3/1, Selector4/1, state_est/1, Selector1/1, Scope2/5, Sum7/2, MATLAB Function6/1(x_k_kprev)
  Sum7/1 -> MATLAB Function7/2(u)
  Random Number4/1 -> Mux3/2
  MATLAB Function8/1(y) -> Sum5/1
  Random Number3/1 -> Mux3/1
  Constant15/1 -> MATLAB Function10/4(Q)
  MATLAB Function9/1(y) -> Sum7/1
  MATLAB Function10/2(P_knext_knext) -> Unit Delay3/1, P0/1
  Sum8/1 -> MATLAB Function8/2(u)
  MATLAB Function10/1(K_knext) -> MATLAB Function9/1(K)
  Constant9/1 -> MATLAB Function7/1(A)
  Constant13/1 -> MATLAB Function10/2(R)
  Sum6/1 -> MATLAB Function9/2(y_err)
  Constant10/1 -> MATLAB Function8/1(B)
  Mux3/1 -> Sum8/1
  Constant16/1 -> MATLAB Function10/5(G)
  inert_vel/1 -> Selector2/1
  Sum5/1 -> Unit Delay2/1
  p/1 -> Selector/1
  Sum/1 -> Sum6/1
  MATLAB Function6/1(pred) -> Sum6/2
  Constant/1 -> MATLAB Function6/2(C), MATLAB Function10/6(C)
  Mux1/1 -> Sum/1
  Selector2/1 -> Sum8/2
  Mux/1 -> Sum/2
  Random Number2/1 -> Mux/2
  Random Number8/1 -> Mux/3
  Random Number1/1 -> Mux/1
  Compare To Constant/1 -> Switch/2
  Memory1/1 -> Compare To Constant/1
  Unit Delay2/1 -> Switch/1
  Ramp/1 -> Memory1/1
  x_0_hat_xy/1 -> Switch/3
  Memory2/1 -> Compare To Constant1/1
  Ramp1/1 -> Memory2/1
  Compare To Constant1/1 -> Switch1/2
  Switch1/1 -> MATLAB Function10/1(P_k_k)
  Unit Delay3/1 -> Switch1/1
  P0_xy/1 -> Switch1/3
  Selector3/1 -> psi_est/1
  Constant12/1 -> Scope2/4, Sum8/3
  Selector/1 -> Mux1/1, Scope2/1
  Selector1/1 -> xy_est/1
  Selector4/1 -> v_c_xy/1
  psi/1 -> Derivative/1, Scope2/2, Mux1/2
  Derivative/1 -> Scope2/3

== system simulation_environment_thesis/PHASE 2.24/z, v_c_z estimate from DEPTH measurement ==
  note: v
  note: u_k = v_m_k
  note: z_m
  p : Inport
  x_0_hat_z : Inport  Port=2
  P0_z : Inport  Port=3
  Compare To Constant : simulink/Logic and Bit Operations/Compare To Constant  relop=>  const=0  OutDataTypeStr=boolean
  Compare To Constant1 : simulink/Logic and Bit Operations/Compare To Constant  relop=>  const=0  OutDataTypeStr=boolean
  Constant10 : Constant  Value=Az1
  Constant11 : Constant  Value=Bz1
  Constant12 : Constant  Value=Cz1  VectorParams1D=off
  Constant13 : Constant  Value=const_current(3,1)
  Constant14 : Constant  Value=Rz1
  Constant15 : Constant  Value=Az1
  Constant16 : Constant  Value=Qz1
  Constant17 : Constant  Value=Gz1
  Constant9 : Constant  Value=Cz1  VectorParams1D=off
  Demux : Demux  Outputs=2
  Derivative1 : Derivative
  MATLAB Function1 : MATLAB Function  TreatAsAtomicUnit=on
  MATLAB Function6 : MATLAB Function  TreatAsAtomicUnit=on
  MATLAB Function7 : MATLAB Function  TreatAsAtomicUnit=on
  MATLAB Function8 : MATLAB Function  TreatAsAtomicUnit=on
  MATLAB Function9 : MATLAB Function  TreatAsAtomicUnit=on
  Memory1 : Memory
  Memory2 : Memory
  Ramp : simulink/Sources/Ramp  slope=1  start=0  InitialOutput=0
  Ramp1 : simulink/Sources/Ramp  slope=1  start=0  InitialOutput=0
  Random Number1 : RandomNumber  Variance=var_vm_z^2  Seed=256526757  SampleTime=dt
  Scope2 : Scope  NumInputPorts=3
  Selector1 : Selector  InputPortWidth=3  IndexOptions=Index vector (dialog)  Indices=[3]  OutputSizes=1
  Sum5 : Sum  Inputs=|++
  Sum6 : Sum  Inputs=|+-
  Sum7 : Sum  Inputs=|++
  Sum8 : Sum  Inputs=|++-
  Sum9 : Sum  Inputs=|++
  Switch : Switch  Criteria=u2 > Threshold  SaturateOnIntegerOverflow=off  InputSameDT=off
  Switch1 : Switch  Criteria=u2 > Threshold  SaturateOnIntegerOverflow=off  InputSameDT=off
  Unit Delay2 : UnitDelay  SampleTime=-1  HasFrameUpgradeWarning=on
  Unit Delay3 : UnitDelay  InitialCondition=alpha_z_1*eye(2)  SampleTime=-1  HasFrameUpgradeWarning=on
  eta3 : RandomNumber  Variance=eta_z_1^2  Seed=1250127822  SampleTime=dt
  z_est : Outport
  state_est : Outport  Port=2
  P0 : Outport  Port=3
  wires:
  Derivative1/1 -> Sum8/2
  Constant11/1 -> MATLAB Function7/1(B)
  Selector1/1 -> Derivative1/1, Scope2/1, Sum9/1
  Constant16/1 -> MATLAB Function9/5(Q)
  Demux/1 -> z_est/1
  MATLAB Function9/2(P_k_k) -> Unit Delay3/1, P0/1
  Constant14/1 -> MATLAB Function9/3(R)
  Unit Delay2/1 -> state_est/1, Demux/1, Scope2/2, Sum7/2, MATLAB Function1/2(u)
  Constant12/1 -> MATLAB Function9/2(C)
  MATLAB Function1/1(y) -> Sum6/2
  MATLAB Function6/1(y) -> Sum5/2
  Sum7/1 -> MATLAB Function6/2(u)
  Random Number1/1 -> Sum8/1
  Sum6/1 -> MATLAB Function8/2(u)
  Constant9/1 -> MATLAB Function1/1(C)
  MATLAB Function9/1(K_k) -> MATLAB Function8/1(K)
  Sum8/1 -> MATLAB Function7/2(u)
  Constant13/1 -> Sum8/3, Scope2/3
  MATLAB Function7/1(y) -> Sum5/1
  Sum9/1 -> Sum6/1
  Constant10/1 -> MATLAB Function6/1(A)
  MATLAB Function8/1(y) -> Sum7/1
  Constant15/1 -> MATLAB Function9/4(A)
  Constant17/1 -> MATLAB Function9/6(G)
  eta3/1 -> Sum9/2
  p/1 -> Selector1/1
  Memory1/1 -> Compare To Constant/1
  Ramp/1 -> Memory1/1
  x_0_hat_z/1 -> Switch/3
  Compare To Constant/1 -> Switch/2
  Switch/1 -> Unit Delay2/1
  Sum5/1 -> Switch/1
  Compare To Constant1/1 -> Switch1/2
  Unit Delay3/1 -> Switch1/1
  Ramp1/1 -> Memory2/1
  Memory2/1 -> Compare To Constant1/1
  P0_z/1 -> Switch1/3
  Switch1/1 -> MATLAB Function9/1("P_k_k ")

== system simulation_environment_thesis/PHASE 3&4  /PHASE  3 ==
  ship_psi_auv : Inport
  ship_p_auv : Inport  Port=2
  ship_p_ds : Inport  Port=3
  Enable : EnablePort
  CONTROLLER : Subsystem
  Constant : Constant  Value=0
  Data Store Read : DataStoreRead  DataStoreName=x_hat_23_4  IndexOptions=Index vector (dialog)  Indices=1  OutputSizes=1
  Data Store Read1 : DataStoreRead  DataStoreName=P0_23_4  IndexOptions=Index vector (dialog)  Indices=1  OutputSizes=1
  Data Store Write : DataStoreWrite  DataStoreName=x_hat_34_4  IndexOptions=Index vector (dialog)  Indices=1  OutputSizes=1
  Data Store Write1 : DataStoreWrite  DataStoreName=P0_34_4  IndexOptions=Index vector (dialog)  Indices=1  OutputSizes=1
  MATLAB Function : MATLAB Function  TreatAsAtomicUnit=on
  MATLAB Function1 : MATLAB Function  TreatAsAtomicUnit=on
  REFERENCE GENERATOR : Subsystem
  Selector1 : Selector  InputPortWidth=4  IndexOptions=Index vector (dialog)  Indices=[1 2 3]  OutputSizes=1
  Selector2 : Selector  InputPortWidth=3  IndexOptions=Index vector (dialog)  Indices=[3]  OutputSizes=1
  Selector3 : Selector  InputPortWidth=3  IndexOptions=Index vector (dialog)  Indices=[1 2]  OutputSizes=1
  Selector4 : Selector  InputPortWidth=8  IndexOptions=Index vector (dialog)  Indices=[1 2 7 8 4 5]  OutputSizes=1
  Selector5 : Selector  InputPortWidth=8  IndexOptions=Index vector (dialog)  Indices=[3 6]  OutputSizes=1
  Sum : Sum  Inputs=|+-
  TAU : Scope
  To Workspace : ToWorkspace  VariableName=switchposition23  SampleTime=-1
  dynamic + kinematic models1 : Subsystem
  "p, v_c, psi, r (3D) estimate, from range, v_c, psi, z measurement" : Subsystem
  v error (robot frame) : Scope
  yaw_err : Scope
  z_err : Scope
  speed body : Outport
  s_p_auv : Outport  Port=2
  s_psi_auv : Outport  Port=3
  ds_p_auv : Outport  Port=4
  state_est_plane : Outport  Port=5
  state_est_z : Outport  Port=6
  z_ err : Outport  Port=7
  yaw_error : Outport  Port=8
  error speed : Outport  Port=9
  wires:
  CONTROLLER/1(tau) -> dynamic + kinematic models1/1(tau), TAU/1
  dynamic + kinematic models1/1(error speed) -> CONTROLLER/1(v_err), error speed/1, v error (robot frame)/1
  MATLAB Function/1(ds_p_auv) -> dynamic + kinematic models1/5(ds_p_auv)
  ship_psi_auv/1 -> dynamic + kinematic models1/3(last_yaw__from_ship)
  ship_p_ds/1 -> MATLAB Function1/2(ship_p_ds), MATLAB Function/2(ship_p_ds)
  MATLAB Function1/1(ship_p_auv) -> s_p_auv/1
  Sum/1 -> CONTROLLER/4(z_err), z_ err/1, z_err/1
  Constant/1 -> Sum/1
  REFERENCE GENERATOR/1(yaw_error) -> CONTROLLER/2(psi_err), yaw_err/1, yaw_error/1
  Selector2/1 -> Sum/2
  "p, v_c, psi, r (3D) estimate, from range, v_c, psi, z measurement"/1(xyz_est) -> Selector2/1, Selector3/1
  "p, v_c, psi, r (3D) estimate, from range, v_c, psi, z measurement"/2(psi_est) -> dynamic + kinematic models1/4(yaw_est), REFERENCE GENERATOR/2(yaw_est)
  "p, v_c, psi, r (3D) estimate, from range, v_c, psi, z measurement"/3(v_c_xyz) -> REFERENCE GENERATOR/3(v_c)
  Selector3/1 -> REFERENCE GENERATOR/1(eta)
  dynamic + kinematic models1/2(v_tot ds) -> "p, v_c, psi, r (3D) estimate, from range, v_c, psi, z measurement"/2(inert_vel)
  Selector1/1 -> MATLAB Function1/1(ds_p_auv), ds_p_auv/1, "p, v_c, psi, r (3D) estimate, from range, v_c, psi, z measurement"/1(p)
  Data Store Read/1 -> "p, v_c, psi, r (3D) estimate, from range, v_c, psi, z measurement"/4(x_0_hat)
  Data Store Read1/1 -> "p, v_c, psi, r (3D) estimate, from range, v_c, psi, z measurement"/5(P0)
  "p, v_c, psi, r (3D) estimate, from range, v_c, psi, z measurement"/5(P0_out) -> Data Store Write1/1
  REFERENCE GENERATOR/2(ref_speed) -> dynamic + kinematic models1/2(ref speed), CONTROLLER/3(speed_0)
  ship_p_auv/1 -> To Workspace/1, MATLAB Function/1(ship_p_auv)
  "p, v_c, psi, r (3D) estimate, from range, v_c, psi, z measurement"/4(state_est) -> Data Store Write/1, Selector5/1, Selector4/1
  Selector4/1 -> state_est_plane/1
  Selector5/1 -> state_est_z/1
  dynamic + kinematic models1/3(p) -> Selector1/1
  dynamic + kinematic models1/4(yaw) -> "p, v_c, psi, r (3D) estimate, from range, v_c, psi, z measurement"/3(psi), s_psi_auv/1
  dynamic + kinematic models1/5(body speed) -> speed body/1

== system simulation_environment_thesis/PHASE 3&4  /PHASE  4 ==
  ship_psi_auv : Inport
  ship_p_auv : Inport  Port=2
  ship_p_ds : Inport  Port=3
  direct_to_4 : Inport  Port=4
  Enable : EnablePort
  CONTROLLER4 : Subsystem
  Constant : Constant  Value=0
  Constant3 : Constant  Value=norm(speed_0)
  Constant5 : Constant  Value=[0; 0]  VectorParams1D=off
  Constant7 : Constant  Value=NED_psi_ds + pi
  EKF INIT : Subsystem
  MATLAB Function : MATLAB Function  TreatAsAtomicUnit=on
  MATLAB Function1 : MATLAB Function  TreatAsAtomicUnit=on
  MATLAB Function11 : MATLAB Function  TreatAsAtomicUnit=on
  MATLAB Function6 : MATLAB Function  TreatAsAtomicUnit=on
  MATLAB Function9 : MATLAB Function  TreatAsAtomicUnit=on
  Memory : Memory
  REFERENCE GENERATOR : Subsystem
  Scope9 : Scope
  Selector1 : Selector  InputPortWidth=4  IndexOptions=Index vector (dialog)  Indices=[1 2 3]  OutputSizes=1
  Selector2 : Selector  InputPortWidth=3  IndexOptions=Index vector (dialog)  Indices=[1 2]  OutputSizes=1
  Selector3 : Selector  InputPortWidth=3  IndexOptions=Index vector (dialog)  Indices=[3]  OutputSizes=1
  Selector4 : Selector  InputPortWidth=3  IndexOptions=Index vector (dialog)  Indices=[1 2]  OutputSizes=1
  Selector6 : Selector  InputPortWidth=8  IndexOptions=Index vector (dialog)  Indices=[1 2 7 8 4 5]  OutputSizes=1
  Selector7 : Selector  InputPortWidth=8  IndexOptions=Index vector (dialog)  Indices=[3 6]  OutputSizes=1
  Stop Simulation : Stop
  Sum : Sum  Inputs=|+-
  Sum4 : Sum  Inputs=|+-
  TAU : Scope
  To Workspace : ToWorkspace  VariableName=switchposition34  SampleTime=-1
  To Workspace1 : ToWorkspace  VariableName=docked  SampleTime=-1
  Unit Delay : UnitDelay  SampleTime=-1  HasFrameUpgradeWarning=on
  dynamic + kinematic models1 : Subsystem
  "p, v_c, psi, r (3D) estimate, from range, v_c, psi, z measurement" : Subsystem
  v error (robot frame)5 : Scope
  z_err : Scope
  speed body : Outport
  s_p_auv : Outport  Port=2
  s_psi_auv : Outport  Port=3
  docked : Outport  Port=4
  state_est_plane : Outport  Port=5
  state_est_z : Outport  Port=6
  ds_p_auv : Outport  Port=7
  z_ err : Outport  Port=8
  angle : Outport  Port=9
  error speed : Outport  Port=10
  wires:
  CONTROLLER4/1(tau) -> dynamic + kinematic models1/1(tau), TAU/1
  dynamic + kinematic models1/1(error speed) -> error speed/1, CONTROLLER4/1(v_err), v error (robot frame)5/1
  MATLAB Function/1(ds_p_auv) -> dynamic + kinematic models1/5(ds_p_auv)
  ship_psi_auv/1 -> dynamic + kinematic models1/3(last_yaw__from_ship)
  ship_p_ds/1 -> MATLAB Function/2(ship_p_ds), MATLAB Function1/2(ship_p_ds)
  MATLAB Function1/1(ship_p_auv) -> s_p_auv/1
  Selector3/1 -> Sum/2
  Sum/1 -> z_ err/1, CONTROLLER4/4(z_err), z_err/1
  Constant/1 -> Sum/1
  dynamic + kinematic models1/2(v_tot ds) -> "p, v_c, psi, r (3D) estimate, from range, v_c, psi, z measurement"/2(inert_vel)
  Sum4/1 -> MATLAB Function6/1("angle ")
  MATLAB Function6/1(angle) -> Scope9/1, angle/1, CONTROLLER4/2(psi_err)
  Unit Delay/1 -> dynamic + kinematic models1/2(ref speed), CONTROLLER4/3(speed_0)
  "p, v_c, psi, r (3D) estimate, from range, v_c, psi, z measurement"/2(psi_est) -> dynamic + kinematic models1/4(yaw_est), Sum4/2
  Selector1/1 -> "p, v_c, psi, r (3D) estimate, from range, v_c, psi, z measurement"/1(p), MATLAB Function1/1(ds_p_auv), ds_p_auv/1
  ship_p_auv/1 -> To Workspace/1, MATLAB Function/1(ship_p_auv)
  Constant5/1 -> MATLAB Function9/2(ds_p_ds)
  Constant7/1 -> MATLAB Function11/1("angle "), Sum4/1
  MATLAB Function9/1(docked) -> Stop Simulation/1, docked/1, To Workspace1/1, Memory/1
  Memory/1 -> MATLAB Function9/3("docked ")
  "p, v_c, psi, r (3D) estimate, from range, v_c, psi, z measurement"/3(v_c_xyz) -> Selector2/1
  "p, v_c, psi, r (3D) estimate, from range, v_c, psi, z measurement"/4(state_est) -> Selector7/1, Selector6/1
  Selector6/1 -> state_est_plane/1
  Selector7/1 -> state_est_z/1
  Selector4/1 -> MATLAB Function9/1(ds_p_auv), REFERENCE GENERATOR/3(ds_p_auv)
  "p, v_c, psi, r (3D) estimate, from range, v_c, psi, z measurement"/1(xyz_est) -> Selector4/1, REFERENCE GENERATOR/2(eta), Selector3/1
  ? -> ?
  MATLAB Function11/1(angle) -> REFERENCE GENERATOR/1(NED_psi_dock)
  Constant3/1 -> REFERENCE GENERATOR/4(v_tot_des)
  Selector2/1 -> REFERENCE GENERATOR/5(v_c)
  REFERENCE GENERATOR/1(v_blend) -> Unit Delay/1
  direct_to_4/1 -> EKF INIT/1(direct_to_4)
  EKF INIT/1(Out1) -> "p, v_c, psi, r (3D) estimate, from range, v_c, psi, z measurement"/4(x_0_hat)
  EKF INIT/2(Out2) -> "p, v_c, psi, r (3D) estimate, from range, v_c, psi, z measurement"/5(P0)
  dynamic + kinematic models1/3(p) -> Selector1/1
  dynamic + kinematic models1/5(body speed) -> speed body/1
  dynamic + kinematic models1/4(yaw) -> s_psi_auv/1, "p, v_c, psi, r (3D) estimate, from range, v_c, psi, z measurement"/3(psi)

== system simulation_environment_thesis/PHASE 3&4  /Subsystem3 ==
  num : Inport
  Enable : EnablePort
  MATLAB Function7 : MATLAB Function  TreatAsAtomicUnit=on
  out : Outport
  wires:
  num/1 -> MATLAB Function7/1(num)
  MATLAB Function7/1(out) -> out/1

== system simulation_environment_thesis/PHASE 3&4  /end phase 3 ==
  note: the DS is in the origin
      | of the DS frame
  ds_p_auv : Inport
  Enable : EnablePort
  # of pings : MATLAB Function  TreatAsAtomicUnit=on
  Constant : Constant  Value=NED_psi_ds
  Constant13 : Constant  Value=[0; 0]  VectorParams1D=off
  Constant8 : Constant  Value=delta_FOV
  num_of_pings : Outport
  wires:
  Constant8/1 -> # of pings/2(delta)
  ds_p_auv/1 -> # of pings/4(ds_p_auv)
  # of pings/1(num_of_pings) -> num_of_pings/1
  Constant13/1 -> # of pings/3(ds_p_ds)
  Constant/1 -> # of pings/1(alpha_ds)

== system simulation_environment_thesis/PHASE 3&4  /logic to recognize where i am, based on the # of pings ==
  note: se out è 1 --> phase 4
      | se out è 0 --> phase 3
  num : Inport
  MATLAB Function6 : MATLAB Function  TreatAsAtomicUnit=on
  out : Outport
  wires:
  MATLAB Function6/1(out) -> out/1
  num/1 -> MATLAB Function6/1(num)

== system simulation_environment_thesis/PHASE 3&4  /simulator of pings from sensor ==
  ds_p_auv : Inport
  stop_flag : Inport  Port=2
  # of pings : MATLAB Function  TreatAsAtomicUnit=on
  Constant : Constant  Value=NED_psi_ds
  Constant13 : Constant  Value=[0; 0]  VectorParams1D=off
  Constant8 : Constant  Value=delta_FOV
  # OF PINGS : Outport
  wires:
  Constant/1 -> # of pings/1(alpha_ds)
  # of pings/1(num_of_pings) -> # OF PINGS/1
  Constant13/1 -> # of pings/3(ds_p_ds)
  Constant8/1 -> # of pings/2(delta)
  ds_p_auv/1 -> # of pings/4(ds_p_auv)
  stop_flag/1 -> # of pings/5(stop_flag)
